simulink model "simulation_environment_thesis"  (R2024b)
settings: Solver=VariableStepAuto  StartTime=0.0  StopTime=3200  RelTol=1e-3
values from neighbouring files (symbols this model uses):
  A = [1, dt; 0, 1]    [estimate_and_control_fully_actuated_with_current.m]
  A1 = [1, 0, 0, 0, dt, 0 ... (first row only)    [estimate_and_control_fully_actuated_with_current.m]
  alpha = 10    [estimate_and_control_fully_actuated_with_current.m]
  alpha_xy_1 = 1    [estimate_and_control_fully_actuated_with_current.m]
  alpha_z_1 = 5    [estimate_and_control_fully_actuated_with_current.m]
  Az1 = [1, dt ... (first row only)    [estimate_and_control_fully_actuated_with_current.m]
  B = [dt; 0]    [estimate_and_control_fully_actuated_with_current.m]
  B1 = [dt, 0 ... (first row only)    [estimate_and_control_fully_actuated_with_current.m]
  Bz1 = [dt ... (first row only)    [estimate_and_control_fully_actuated_with_current.m]
  C1 = [1, 0, 0, 0, 0, 0 ... (first row only)    [estimate_and_control_fully_actuated_with_current.m]
  circumference_x = min_dist_from_ds*cos(theta)    [estimate_and_control_fully_actuated_with_current.m]
  circumference_y = min_dist_from_ds*sin(theta)    [estimate_and_control_fully_actuated_with_current.m]
  const_current = [-r; -s; 0; 0]    [estimate_and_control_fully_actuated_with_current.m]
  csi_psi_1 = 0.0003    [estimate_and_control_fully_actuated_with_current.m]
  csi_r_1 = 0.0003    [estimate_and_control_fully_actuated_with_current.m]
  csi_vcx_1 = 0.0003    [estimate_and_control_fully_actuated_with_current.m]
  csi_vcy_1 = 0.0003    [estimate_and_control_fully_actuated_with_current.m]
  csi_vcz_1 = 0.005    [estimate_and_control_fully_actuated_with_current.m]
  csi_x_1 = 0.003    [estimate_and_control_fully_actuated_with_current.m]
  csi_y_1 = 0.003    [estimate_and_control_fully_actuated_with_current.m]
  csi_z_1 = 0.005    [estimate_and_control_fully_actuated_with_current.m]
  Cz1 = [1, 0]    [estimate_and_control_fully_actuated_with_current.m]
  d = 1    [estimate_and_control_fully_actuated_with_current.m]
  delta_FOV = pi/7    (= 0.448798950513)    [estimate_and_control_fully_actuated_with_current.m]
  desired_speed = speed_0    [estimate_and_control_fully_actuated_with_current.m]
  dt = 1/Tsim    (= 0.005)    [estimate_and_control_fully_actuated_with_current.m]
  eta1 = deg2rad(0.5)    [estimate_and_control_fully_actuated_with_current.m]
  eta2 = deg2rad(0.5)    [estimate_and_control_fully_actuated_with_current.m]
  eta_psi_1 = 0.003    [estimate_and_control_fully_actuated_with_current.m]
  eta_x_1 = 0.03    [estimate_and_control_fully_actuated_with_current.m]
  eta_y_1 = 0.03    [estimate_and_control_fully_actuated_with_current.m]
  eta_z_1 = 0.005    [estimate_and_control_fully_actuated_with_current.m]
  G = dt*eye(2)    [estimate_and_control_fully_actuated_with_current.m]
  G1 = dt*eye(6)    [estimate_and_control_fully_actuated_with_current.m]
  Gz1 = dt*eye(2)    [estimate_and_control_fully_actuated_with_current.m]
  left_reference = L_x*[cos(NED_psi_ds); sin(NED_psi_ds); 0] + L_y_l*[-sin(NED_psi_ds); cos(NED_psi_ds); 0]    [estimate_and_control_fully_actuated_with_current.m]
  max_dist_from_ship = 60    [estimate_and_control_fully_actuated_with_current.m]
  min_dist_from_ds = 45    [estimate_and_control_fully_actuated_with_current.m]
  mu = -5    [estimate_and_control_fully_actuated_with_current.m]
  NED_psi_ds = -pi/2    (= -1.57079632679)    [estimate_and_control_fully_actuated_with_current.m]
  p_b1 = [-90; 90; 5]    [estimate_and_control_fully_actuated_with_current.m]
  position_t_0 = [0; 0; 0; 0]    [estimate_and_control_fully_actuated_with_current.m]
  psi_P = linspace(0,2*pi,360)'    [estimate_and_control_fully_actuated_with_current.m]
  Q = [csi1_kf^2, 0 ... (first row only)    [estimate_and_control_fully_actuated_with_current.m]
  Q1 = [csi_x_1_kf^2, 0, 0, 0, 0, 0 ... (first row only)    [estimate_and_control_fully_actuated_with_current.m]
  Qz1 = [csi_z_1_kf^2, 0 ... (first row only)    [estimate_and_control_fully_actuated_with_current.m]
  R = [eta1_kf^2, 0 ... (first row only)    [estimate_and_control_fully_actuated_with_current.m]
  R1 = [eta_x_1_kf^2, 0, 0 ... (first row only)    [estimate_and_control_fully_actuated_with_current.m]
  right_reference = L_x*[cos(NED_psi_ds); sin(NED_psi_ds); 0] + L_y_r*[sin(NED_psi_ds); -cos(NED_psi_ds); 0]    [estimate_and_control_fully_actuated_with_current.m]
  Rz1 = eta_z_1_kf^2    (= 1e-06)    [estimate_and_control_fully_actuated_with_current.m]
  speed_0 = [0.1, 0.1, 0, 0]'    [estimate_and_control_fully_actuated_with_current.m]
  tau_heave_sat = 30    [estimate_and_control_fully_actuated_with_current.m]
  tau_surge_sat = 10    [estimate_and_control_fully_actuated_with_current.m]
  tau_sway_sat = 30    [estimate_and_control_fully_actuated_with_current.m]
  tau_yaw_sat = 0.1    [estimate_and_control_fully_actuated_with_current.m]
  var_vm_x = 0.001    [estimate_and_control_fully_actuated_with_current.m]
  var_vm_y = 0.001    [estimate_and_control_fully_actuated_with_current.m]
  var_vm_z = 0.001    [estimate_and_control_fully_actuated_with_current.m]
undefined symbols (not defined in the file or in its folder): A2, alpha2, B2, csi1_2, csi2_2, csi3_2, csi4_2, csi5_2, csi6_2, csi7_2, csi8_2, eta1_2, eta2_2, eta3_2, eta4_2, eta5_2, eta6_2, G2, Q2, R2
libraries: simulink x60, simulink_extras x5
(part 2 of 16)

== system simulation_environment_thesis/PHASE 2.22 ==
  note: i know the beacon is my new
      | reference frame, so (0,0,0)
  note: i know the beacon is my new
      | reference frame, so (0,0,0)
  note: true position to plot the data in matlab
  note: physical data to be sent to
      | the range-measurement simulator
  note: ship_psi_auv
  note: ok, trasformazione coerente
  note: ok cominciano esattamente dalla flag
  ship_psi_auv : Inport
  ship_p_auv : Inport  Port=2
  ship_p_ds : Inport  Port=3
  Enable : EnablePort
  CONTROLLER4 : Subsystem
  Constant : Constant  Value=0
  Constant1 : Constant  Value=min_dist_from_ds
  Constant9 : Constant  Value=desired_speed
  Data Store Read : DataStoreRead  DataStoreName=x_hat_12_xy_3  IndexOptions=Index vector (dialog)  Indices=1  OutputSizes=1
  Data Store Read1 : DataStoreRead  DataStoreName=x_hat_12_z_3  IndexOptions=Index vector (dialog)  Indices=1  OutputSizes=1
  Data Store Read2 : DataStoreRead  DataStoreName=P0_12_xy_3  IndexOptions=Index vector (dialog)  Indices=1  OutputSizes=1
  Data Store Read3 : DataStoreRead  DataStoreName=P0_12_z_3  IndexOptions=Index vector (dialog)  Indices=1  OutputSizes=1
  Data Store Write : DataStoreWrite  DataStoreName=x_hat_23_3  IndexOptions=Index vector (dialog)  Indices=1  OutputSizes=1
  Data Store Write1 : DataStoreWrite  DataStoreName=P0_23_3  IndexOptions=Index vector (dialog)  Indices=1  OutputSizes=1
  MATLAB Function : MATLAB Function  TreatAsAtomicUnit=on
  MATLAB Function1 : MATLAB Function  TreatAsAtomicUnit=on
  MATLAB Function2 : MATLAB Function  TreatAsAtomicUnit=on
  MATLAB Function7 : MATLAB Function  TreatAsAtomicUnit=on
  Memory2 : Memory
  Scope{,1..3} (4 alike) : Scope
  Selector1 : Selector  InputPortWidth=4  IndexOptions=Index vector (dialog)  Indices=[1 2 3]  OutputSizes=1
  Selector2 : Selector  InputPortWidth=3  IndexOptions=Index vector (dialog)  Indices=[3]  OutputSizes=1
  Selector3 : Selector  InputPortWidth=3  IndexOptions=Index vector (dialog)  Indices=[1 2]  OutputSizes=1
  Selector4 : Selector  InputPortWidth=8  IndexOptions=Index vector (dialog)  Indices=[1 2 7 8 4 5]  OutputSizes=1
  Selector5 : Selector  InputPortWidth=8  IndexOptions=Index vector (dialog)  Indices=[3 6]  OutputSizes=1
  Sum : Sum  Inputs=|+-
  Sum1 : Sum  Inputs=|+-
  TAU : Scope
  To Workspace : ToWorkspace  VariableName=switchposition12  SampleTime=-1
  dynamic + kinematic models1 : Subsystem
  new origin : Constant  Value=[0; 0]  VectorParams1D=off
  "p, v_c, psi, r (3D) estimate, from RANGE, v_c, PSI measurement" : Subsystem
  v error (robot frame)5 : Scope
  yaw_err3 : Scope
  z_err : Scope
  s_p_auv : Outport
  s_psi_auv : Outport  Port=2
  stop_flag_2 : Outport  Port=3
  ds_p_auv : Outport  Port=4
  body speed : Outport  Port=5
  state_est_plane : Outport  Port=6
  state_est_z : Outport  Port=7
  angle : Outport  Port=8
  error speed : Outport  Port=9
  z_ err : Outport  Port=10
  wires:
  "p, v_c, psi, r (3D) estimate, from RANGE, v_c, PSI measurement"/2(psi_est) -> dynamic + kinematic models1/4(yaw_est), Sum1/2
  Constant9/1 -> dynamic + kinematic models1/2(ref speed), MATLAB Function7/5(speed), CONTROLLER4/3(speed_0)
  CONTROLLER4/1(tau) -> dynamic + kinematic models1/1(tau), TAU/1
  dynamic + kinematic models1/1(error speed) -> CONTROLLER4/1(v_err), error speed/1, v error (robot frame)5/1
  ship_psi_auv/1 -> dynamic + kinematic models1/3(last_yaw__from_ship)
  ship_p_ds/1 -> MATLAB Function1/2(ship_p_ds), MATLAB Function/2(ship_p_ds)
  dynamic + kinematic models1/3(p) -> Selector1/1
  MATLAB Function1/1(ship_p_auv) -> s_p_auv/1
  dynamic + kinematic models1/4(yaw) -> s_psi_auv/1, "p, v_c, psi, r (3D) estimate, from RANGE, v_c, PSI measurement"/3(psi)
  ship_p_auv/1 -> To Workspace/1, MATLAB Function/1(ship_p_auv)
  Sum1/1 -> MATLAB Function2/1("angle ")
  dynamic + kinematic models1/2(v_tot ds) -> "p, v_c, psi, r (3D) estimate, from RANGE, v_c, PSI measurement"/2(inert_vel)
  Sum/1 -> CONTROLLER4/4(z_err), z_ err/1, z_err/1
  Constant/1 -> Sum/1
  new origin/1 -> MATLAB Function7/4(beacon)
  Constant1/1 -> MATLAB Function7/6(threshold)
  "p, v_c, psi, r (3D) estimate, from RANGE, v_c, PSI measurement"/3(v_c_xyz) -> MATLAB Function7/2(v_c)
  MATLAB Function7/1(yaw_desired) -> Scope3/1, Sum1/1
  MATLAB Function7/2(stop_flag) -> Memory2/1, Scope/1, stop_flag_2/1
  MATLAB Function7/3(ds_dist) -> Scope2/1
  MATLAB Function2/1(angle) -> angle/1, CONTROLLER4/2(psi_err), yaw_err3/1
  Memory2/1 -> MATLAB Function7/1("stop_flag ")
  "p, v_c, psi, r (3D) estimate, from RANGE, v_c, PSI measurement"/1(xyz_est) -> Selector2/1, Selector3/1
  Selector2/1 -> Sum/2
  Selector3/1 -> MATLAB Function7/3(p)
  dynamic + kinematic models1/5(body speed) -> body speed/1
  MATLAB Function/1(ds_p_auv) -> dynamic + kinematic models1/5(ds_p_auv), Scope1/1
  Selector1/1 -> ds_p_auv/1, MATLAB Function1/1(ds_p_auv), "p, v_c, psi, r (3D) estimate, from RANGE, v_c, PSI measurement"/1(p)
  Data Store Read/1 -> "p, v_c, psi, r (3D) estimate, from RANGE, v_c, PSI measurement"/4(x_0_hat_xy)
  Data Store Read1/1 -> "p, v_c, psi, r (3D) estimate, from RANGE, v_c, PSI measurement"/5(x_0_hat_z)
  Data Store Read2/1 -> "p, v_c, psi, r (3D) estimate, from RANGE, v_c, PSI measurement"/6(P0_xy)
  Data Store Read3/1 -> "p, v_c, psi, r (3D) estimate, from RANGE, v_c, PSI measurement"/7(P0_z)
  "p, v_c, psi, r (3D) estimate, from RANGE, v_c, PSI measurement"/5(P023) -> Data Store Write1/1
  Selector5/1 -> state_est_z/1
  Selector4/1 -> state_est_plane/1
  "p, v_c, psi, r (3D) estimate, from RANGE, v_c, PSI measurement"/4(state_est) -> Selector5/1, Selector4/1, Data Store Write/1

== system simulation_environment_thesis/PHASE 2.24 ==
  note: ds_psi_auv
  note: ok, trasformazione coerente
  note: ok cominciano esattamente dalla flag
  ship_psi_auv : Inport
  ship_p_auv : Inport  Port=2
  ship_p_ds : Inport  Port=3
  Enable : EnablePort
  CONTROLLER4 : Subsystem
  Constant : Constant  Value=0
  Constant1 : Constant  Value=min_dist_from_ds
  Constant9 : Constant  Value=desired_speed
  Data Store Read : DataStoreRead  DataStoreName=x_hat_12_xy_1  IndexOptions=Index vector (dialog)  Indices=1  OutputSizes=1
  Data Store Read1 : DataStoreRead  DataStoreName=P0_12_xy_1  IndexOptions=Index vector (dialog)  Indices=1  OutputSizes=1
  Data Store Read2 : DataStoreRead  DataStoreName=x_hat_12_z_1  IndexOptions=Index vector (dialog)  Indices=1  OutputSizes=1
  Data Store Read3 : DataStoreRead  DataStoreName=P0_12_z_1  IndexOptions=Index vector (dialog)  Indices=1  OutputSizes=1
  Data Store Write : DataStoreWrite  DataStoreName=x_hat_23_xy_1  IndexOptions=Index vector (dialog)  Indices=1  OutputSizes=1
  Data Store Write1 : DataStoreWrite  DataStoreName=P0_23_xy_1  IndexOptions=Index vector (dialog)  Indices=1  OutputSizes=1
  Data Store Write2 : DataStoreWrite  DataStoreName=x_hat_23_z_1  IndexOptions=Index vector (dialog)  Indices=1  OutputSizes=1
  Data Store Write3 : DataStoreWrite  DataStoreName=P0_23_z_1  IndexOptions=Index vector (dialog)  Indices=1  OutputSizes=1
  MATLAB Function : MATLAB Function  TreatAsAtomicUnit=on
  MATLAB Function1 : MATLAB Function  TreatAsAtomicUnit=on
  MATLAB Function2 : MATLAB Function  TreatAsAtomicUnit=on
  MATLAB Function7 : MATLAB Function  TreatAsAtomicUnit=on
  Memory2 : Memory
  Scope1 : Scope
  Scope2 : Scope
  Scope3 : Scope
  Selector1 : Selector  InputPortWidth=4  IndexOptions=Index vector (dialog)  Indices=[1 2 3]  OutputSizes=1
  Sum : Sum  Inputs=|+-
  Sum1 : Sum  Inputs=|+-
  TAU : Scope
  To Workspace : ToWorkspace  VariableName=switchposition12  SampleTime=-1
  dynamic + kinematic models1 : Subsystem
  new origin : Constant  Value=[0; 0]  VectorParams1D=off
  "positon and current estimate, XY PLANE from X, Y, psi measurement" : Subsystem
  v error (robot frame)5 : Scope
  yaw_err3 : Scope
  "z, v_c_z estimate from DEPTH measurement" : Subsystem
  z_err : Scope
  speed body : Outport
  s_p_auv : Outport  Port=2
  s_psi_auv : Outport  Port=3
  stop_flag_2 : Outport  Port=4
  ds_p_auv : Outport  Port=5
  state_est_plane : Outport  Port=6
  state_est_z : Outport  Port=7
  error speed : Outport  Port=8
  angle : Outport  Port=9
  "z_err " : Outport  Port=10
  wires:
  "positon and current estimate, XY PLANE from X, Y, psi measurement"/2(psi_est) -> dynamic + kinematic models1/4(yaw_est), Sum1/2
  Constant9/1 -> dynamic + kinematic models1/2(ref speed), CONTROLLER4/3(speed_0), MATLAB Function7/5(speed)
  CONTROLLER4/1(tau) -> dynamic + kinematic models1/1(tau), TAU/1
  dynamic + kinematic models1/1(error speed) -> error speed/1, CONTROLLER4/1(v_err), v error (robot frame)5/1
  MATLAB Function/1(ds_p_auv) -> dynamic + kinematic models1/5(ds_p_auv), Scope1/1
  ship_psi_auv/1 -> dynamic + kinematic models1/3(last_yaw__from_ship)
  ship_p_ds/1 -> MATLAB Function1/2(ship_p_ds), MATLAB Function/2(ship_p_ds)
  dynamic + kinematic models1/4(p) -> Selector1/1, "positon and current estimate, XY PLANE from X, Y, psi measurement"/1(p)
  MATLAB Function1/1(ship_p_auv) -> s_p_auv/1
  dynamic + kinematic models1/5(yaw) -> s_psi_auv/1, "positon and current estimate, XY PLANE from X, Y, psi measurement"/3(psi)
  ship_p_auv/1 -> To Workspace/1, MATLAB Function/1(ship_p_auv)
  Sum1/1 -> MATLAB Function2/1("angle ")
  dynamic + kinematic models1/2(v_tot ds) -> "positon and current estimate, XY PLANE from X, Y, psi measurement"/2(inert_vel)
  dynamic + kinematic models1/3(body speed) -> speed body/1
  "z, v_c_z estimate from DEPTH measurement"/1(z_est) -> Sum/2
  Sum/1 -> z_err/1, "z_err "/1, CONTROLLER4/4(z_err)
  Constant/1 -> Sum/1
  new origin/1 -> MATLAB Function7/4(beacon)
  Constant1/1 -> MATLAB Function7/6(threshold)
  "positon and current estimate, XY PLANE from X, Y, psi measurement"/3(v_c_xy) -> MATLAB Function7/2(v_c)
  MATLAB Function7/1(yaw_desired) -> Scope3/1, Sum1/1
  MATLAB Function7/2(stop_flag) -> Memory2/1, stop_flag_2/1
  MATLAB Function7/3(ds_dist) -> Scope2/1
  MATLAB Function2/1(angle) -> angle/1, CONTROLLER4/2(psi_err), yaw_err3/1
  Memory2/1 -> MATLAB Function7/1("stop_flag ")
  "positon and current estimate, XY PLANE from X, Y, psi measurement"/1(xy_est) -> MATLAB Function7/3(p)
  Data Store Read/1 -> "positon and current estimate, XY PLANE from X, Y, psi measurement"/4(x_0_hat_xy)
  Data Store Read1/1 -> "positon and current estimate, XY PLANE from X, Y, psi measurement"/5(P0_xy)
  "positon and current estimate, XY PLANE from X, Y, psi measurement"/4(state_est) -> state_est_plane/1, Data Store Write/1
  "positon and current estimate, XY PLANE from X, Y, psi measurement"/5(P0) -> Data Store Write1/1
  Selector1/1 -> ds_p_auv/1, "z, v_c_z estimate from DEPTH measurement"/1(p), MATLAB Function1/1(ds_p_auv)
  "z, v_c_z estimate from DEPTH measurement"/2(state_est) -> state_est_z/1, Data Store Write2/1
  "z, v_c_z estimate from DEPTH measurement"/3(P0) -> Data Store Write3/1
  Data Store Read2/1 -> "z, v_c_z estimate from DEPTH measurement"/2(x_0_hat_z)
  Data Store Read3/1 -> "z, v_c_z estimate from DEPTH measurement"/3(P0_z)

== system simulation_environment_thesis/PHASE 3&4   ==
  note: from phase 2 to either
      | phase 3 or directly phase 4
  note: FASE 4
  ship_p_ds : Inport
  ds_p_auv : Inport  Port=2
  stop_flag : Inport  Port=3
  ship_psi_auv : Inport  Port=4
  ship_p_auv : Inport  Port=5
  Enable : EnablePort
  AND1 : Logic  IconShape=distinctive  AllPortsSameDT=off  OutDataTypeStr=boolean
  AND2 : Logic  IconShape=distinctive  AllPortsSameDT=off  OutDataTypeStr=boolean
  NOT3 : Logic  Operator=NOT  IconShape=distinctive  AllPortsSameDT=off  OutDataTypeStr=boolean
  NOT5 : Logic  Operator=NOT  IconShape=distinctive  AllPortsSameDT=off  OutDataTypeStr=boolean
  NOT6 : Logic  Operator=NOT  IconShape=distinctive  AllPortsSameDT=off  OutDataTypeStr=boolean
  OR : Logic  Operator=OR  IconShape=distinctive  AllPortsSameDT=off  OutDataTypeStr=boolean
  PHASE  3 : Subsystem
  PHASE  4 : Subsystem
  Scope{3..4,10..12} (5 alike) : Scope
  Subsystem3 : Subsystem
  Switch : Switch  Criteria=u2 > Threshold  SaturateOnIntegerOverflow=off  InputSameDT=off
  Switch1 : Switch  Criteria=u2 > Threshold  SaturateOnIntegerOverflow=off  InputSameDT=off
  To Workspace : ToWorkspace  VariableName=pass_by_3  SampleTime=-1
  Unit Delay4 : UnitDelay  InitialCondition=1  SampleTime=-1  HasFrameUpgradeWarning=on
  Unit Delay5 : UnitDelay  InitialCondition=1  SampleTime=-1  HasFrameUpgradeWarning=on
  end phase 3 : Subsystem
  "logic to recognize where i am, based on the # of pings" : Subsystem
  simulator of pings from sensor : Subsystem
  speed body : Outport
  ship_psi_auv1 : Outport  Port=2
  ship_p_auv1 : Outport  Port=3
  speed body1 : Outport  Port=4
  ship_psi_auv2 : Outport  Port=5
  ship_p_auv2 : Outport  Port=6
  out : Outport  Port=7
  out1 : Outport  Port=8
  docked : Outport  Port=9
  ds_p_auv_3 : Outport  Port=10
  state_est_plane4 : Outport  Port=11
  state_est_z3 : Outport  Port=12
  state_est_plane3 : Outport  Port=13
  state_est_z4 : Outport  Port=14
  ds_p_auv4 : Outport  Port=15
  z_ err : Outport  Port=16
  yaw_error : Outport  Port=17
  error speed : Outport  Port=18
  z_ err 4 : Outport  Port=19
  angle 4 : Outport  Port=20
  error speed 4 : Outport  Port=21
  wires:
  Unit Delay5/1 -> AND2/2
  PHASE  3/4(ds_p_auv) -> ds_p_auv_3/1, end phase 3/1(ds_p_auv)
  Subsystem3/1(out) -> out1/1, Scope12/1, OR/1, NOT5/1
  PHASE  4/2(s_p_auv) -> ship_p_auv2/1
  ship_p_ds/1 -> PHASE  4/3(ship_p_ds), PHASE  3/3(ship_p_ds)
  "logic to recognize where i am, based on the # of pings"/1(out) -> PHASE  4/4(direct_to_4), Switch1/2, Switch/2, out/1, NOT3/1, To Workspace/1, Scope4/1, OR/2
  ship_psi_auv/1 -> Switch/1, PHASE  3/1(ship_psi_auv)
  Unit Delay4/1 -> AND1/2
  PHASE  4/4(docked) -> docked/1, NOT6/1, Scope3/1
  simulator of pings from sensor/1(# OF PINGS) -> "logic to recognize where i am, based on the # of pings"/1(num)
  NOT3/1 -> Subsystem3/Enable, end phase 3/Enable, AND1/1
  AND1/1 -> PHASE  3/Enable
  OR/1 -> Scope11/1, AND2/1
  PHASE  3/2(s_p_auv) -> Switch1/3, ship_p_auv1/1
  PHASE  4/3(s_psi_auv) -> ship_psi_auv2/1
  NOT5/1 -> Unit Delay4/1
  PHASE  3/3(s_psi_auv) -> Switch/3, ship_psi_auv1/1
  end phase 3/1(num_of_pings) -> Subsystem3/1(num)
  AND2/1 -> Scope10/1, PHASE  4/Enable
  stop_flag/1 -> simulator of pings from sensor/2(stop_flag)
  ds_p_auv/1 -> simulator of pings from sensor/1(ds_p_auv)
  ship_p_auv/1 -> Switch1/1, PHASE  3/2(ship_p_auv)
  PHASE  4/1(speed body) -> speed body1/1
  PHASE  3/1(speed body) -> speed body/1
  NOT6/1 -> Unit Delay5/1
  Switch/1 -> PHASE  4/1(ship_psi_auv)
  Switch1/1 -> PHASE  4/2(ship_p_auv)
  PHASE  3/5(state_est_plane) -> state_est_plane3/1
  PHASE  3/6(state_est_z) -> state_est_z3/1
  PHASE  4/7(ds_p_auv) -> ds_p_auv4/1
  PHASE  4/6(state_est_z) -> state_est_z4/1
  PHASE  4/5(state_est_plane) -> state_est_plane4/1
  PHASE  3/7(z_ err) -> z_ err/1
  PHASE  3/8(yaw_error) -> yaw_error/1
  PHASE  3/9(error speed) -> error speed/1
  PHASE  4/8(z_ err) -> z_ err 4/1
  PHASE  4/9(angle) -> angle 4/1
  PHASE  4/10(error speed) -> error speed 4/1

== system simulation_environment_thesis/PHASE 3&4.21 ==
  note: from phase 2 to either phase 3 or directly phase 4
  note: FASE 4
  ship_p_ds : Inport
  ds_p_auv : Inport  Port=2
  stop_flag : Inport  Port=3
  ship_psi_auv : Inport  Port=4
  ship_p_auv : Inport  Port=5
  Enable : EnablePort
  AND1 : Logic  IconShape=distinctive  AllPortsSameDT=off  OutDataTypeStr=boolean
  AND2 : Logic  IconShape=distinctive  AllPortsSameDT=off  OutDataTypeStr=boolean
  NOT3 : Logic  Operator=NOT  IconShape=distinctive  AllPortsSameDT=off  OutDataTypeStr=boolean
  NOT5 : Logic  Operator=NOT  IconShape=distinctive  AllPortsSameDT=off  OutDataTypeStr=boolean
  NOT6 : Logic  Operator=NOT  IconShape=distinctive  AllPortsSameDT=off  OutDataTypeStr=boolean
  OR : Logic  Operator=OR  IconShape=distinctive  AllPortsSameDT=off  OutDataTypeStr=boolean
  PHASE  3 : Subsystem
  PHASE  4 : Subsystem
  Scope{3..4,8,10..12} (6 alike) : Scope
  Subsystem3 : Subsystem
  Switch : Switch  Criteria=u2 > Threshold  SaturateOnIntegerOverflow=off  InputSameDT=off
  Switch1 : Switch  Criteria=u2 > Threshold  SaturateOnIntegerOverflow=off  InputSameDT=off
  To Workspace : ToWorkspace  VariableName=pass_by_3  SampleTime=-1
  Unit Delay4 : UnitDelay  InitialCondition=1  SampleTime=-1  HasFrameUpgradeWarning=on
  Unit Delay5 : UnitDelay  InitialCondition=1  SampleTime=-1  HasFrameUpgradeWarning=on
  end phase 3 : Subsystem
  "logic to recognize where i am, based on the # of pings" : Subsystem
  simulator of pings from sensor : Subsystem
  speed body : Outport
  ship_psi_auv1 : Outport  Port=2
  ship_p_auv1 : Outport  Port=3
  speed body1 : Outport  Port=4
  ship_psi_auv2 : Outport  Port=5
  ship_p_auv2 : Outport  Port=6
  out : Outport  Port=7
  out1 : Outport  Port=8
  docked : Outport  Port=9
  state_est_plane_3 : Outport  Port=10
  state_est_z_3 : Outport  Port=11
  state_est_plane_4 : Outport  Port=12
  state_est_z_4 : Outport  Port=13
  ds_p_auv_3 : Outport  Port=14
  ds_p_auv_4 : Outport  Port=15
  "z_err " : Outport  Port=16
  error speed : Outport  Port=17
  yaw_error : Outport  Port=18
  z_err 4 : Outport  Port=19
  angle 4 : Outport  Port=20
  error speed 4 : Outport  Port=21
  wires:
  Unit Delay5/1 -> AND2/2
  PHASE  3/4(ds_p_auv) -> end phase 3/1(ds_p_auv), ds_p_auv_3/1
  Subsystem3/1(out) -> out1/1, Scope12/1, OR/1, NOT5/1
  PHASE  4/2(s_p_auv) -> ship_p_auv2/1
  ship_p_ds/1 -> PHASE  4/3(ship_p_ds), PHASE  3/3(ship_p_ds)
  "logic to recognize where i am, based on the # of pings"/1(out) -> PHASE  4/4(direct_to_4), Switch1/2, Switch/2, out/1, NOT3/1, To Workspace/1, Scope4/1, OR/2
  ship_psi_auv/1 -> Switch/1, PHASE  3/1(ship_psi_auv)
  PHASE  4/4(new_speed_desired) -> Scope8/1
  Unit Delay4/1 -> AND1/2
  PHASE  4/5(docked) -> docked/1, NOT6/1, Scope3/1
  simulator of pings from sensor/1(# OF PINGS) -> "logic to recognize where i am, based on the # of pings"/1(num)
  NOT3/1 -> Subsystem3/Enable, end phase 3/Enable, AND1/1
  AND1/1 -> PHASE  3/Enable
  OR/1 -> Scope11/1, AND2/1
  PHASE  3/2(s_p_auv) -> Switch1/3, ship_p_auv1/1
  PHASE  4/3(s_psi_auv) -> ship_psi_auv2/1
  NOT5/1 -> Unit Delay4/1
  PHASE  3/3(s_psi_auv) -> Switch/3, ship_psi_auv1/1
  end phase 3/1(num_of_pings) -> Subsystem3/1(num)
  AND2/1 -> Scope10/1, PHASE  4/Enable
  stop_flag/1 -> simulator of pings from sensor/2(stop_flag)
  ds_p_auv/1 -> simulator of pings from sensor/1(ds_p_auv)
  ship_p_auv/1 -> Switch1/1, PHASE  3/2(ship_p_auv)
  PHASE  4/1(speed body) -> speed body1/1
  PHASE  3/1(speed body) -> speed body/1
  NOT6/1 -> Unit Delay5/1
  Switch/1 -> PHASE  4/1(ship_psi_auv)
  Switch1/1 -> PHASE  4/2(ship_p_auv)
  PHASE  3/5(state_est_plane) -> state_est_plane_3/1
  PHASE  3/6(state_est_z) -> state_est_z_3/1
  PHASE  4/6(state_est_plane) -> state_est_plane_4/1
  PHASE  4/7(state_est_z) -> state_est_z_4/1
  PHASE  4/8(ds_p_auv_4) -> ds_p_auv_4/1
  PHASE  3/7("z_err ") -> "z_err "/1
  PHASE  3/8(error speed) -> error speed/1
  PHASE  3/9(yaw_error) -> yaw_error/1
  PHASE  4/9(z_ err) -> z_err 4/1
  PHASE  4/10(angle) -> angle 4/1
  PHASE  4/11(error speed) -> error speed 4/1

== system simulation_environment_thesis/PHASE 3&4.22 ==
  note: from phase 2 to either phase 3 or directly phase 4
  note: FASE 4
  ship_p_ds : Inport
  ds_p_auv : Inport  Port=2
  stop_flag : Inport  Port=3
  ship_psi_auv : Inport  Port=4
  ship_p_auv : Inport  Port=5
  Enable : EnablePort
  AND1 : Logic  IconShape=distinctive  AllPortsSameDT=off  OutDataTypeStr=boolean
  AND2 : Logic  IconShape=distinctive  AllPortsSameDT=off  OutDataTypeStr=boolean
  NOT3 : Logic  Operator=NOT  IconShape=distinctive  AllPortsSameDT=off  OutDataTypeStr=boolean
  NOT5 : Logic  Operator=NOT  IconShape=distinctive  AllPortsSameDT=off  OutDataTypeStr=boolean
  NOT6 : Logic  Operator=NOT  IconShape=distinctive  AllPortsSameDT=off  OutDataTypeStr=boolean
  OR : Logic  Operator=OR  IconShape=distinctive  AllPortsSameDT=off  OutDataTypeStr=boolean
  PHASE  3 : Subsystem
  PHASE  4 : Subsystem
  Scope{3..4,8,10..12} (6 alike) : Scope
  Subsystem3 : Subsystem
  Switch : Switch  Criteria=u2 > Threshold  SaturateOnIntegerOverflow=off  InputSameDT=off
  Switch1 : Switch  Criteria=u2 > Threshold  SaturateOnIntegerOverflow=off  InputSameDT=off
  To Workspace : ToWorkspace  VariableName=pass_by_3  SampleTime=-1
  Unit Delay4 : UnitDelay  InitialCondition=1  SampleTime=-1  HasFrameUpgradeWarning=on
  Unit Delay5 : UnitDelay  InitialCondition=1  SampleTime=-1  HasFrameUpgradeWarning=on
  end phase 3 : Subsystem
  "logic to recognize where i am, based on the # of pings" : Subsystem
  simulator of pings from sensor : Subsystem
  speed body : Outport
  ship_psi_auv1 : Outport  Port=2
  ship_p_auv1 : Outport  Port=3
  speed body1 : Outport  Port=4
  ship_psi_auv2 : Outport  Port=5
  ship_p_auv2 : Outport  Port=6
  out : Outport  Port=7
  out1 : Outport  Port=8
  docked : Outport  Port=9
  ds_p_auv_3 : Outport  Port=10
  ds_p_auv4 : Outport  Port=11
  state_est_plane4 : Outport  Port=12
  state_est_z4 : Outport  Port=13
  state_est_plane3 : Outport  Port=14
  state_est_z3 : Outport  Port=15
  yaw_rel_err : Outport  Port=16
  error speed : Outport  Port=17
  yaw_error : Outport  Port=18
  z_ err : Outport  Port=19
  error speed 4 : Outport  Port=20
  angle 4 : Outport  Port=21
  "z_ err 4 " : Outport  Port=22
  wires:
  Unit Delay5/1 -> AND2/2
  PHASE  3/4(ds_p_auv) -> end phase 3/1(ds_p_auv), ds_p_auv_3/1
  Subsystem3/1(out) -> out1/1, Scope12/1, OR/1, NOT5/1
  PHASE  4/2(s_p_auv) -> ship_p_auv2/1
  ship_p_ds/1 -> PHASE  4/3(ship_p_ds), PHASE  3/3(ship_p_ds)
  "logic to recognize where i am, based on the # of pings"/1(out) -> PHASE  4/4(direct_to_4), Switch1/2, Switch/2, out/1, NOT3/1, To Workspace/1, Scope4/1, OR/2
  ship_psi_auv/1 -> Switch/1, PHASE  3/1(ship_psi_auv)
  PHASE  4/4(new_speed_desired) -> Scope8/1
  Unit Delay4/1 -> AND1/2
  PHASE  4/5(docked) -> NOT6/1, Scope3/1, docked/1
  simulator of pings from sensor/1(# OF PINGS) -> "logic to recognize where i am, based on the # of pings"/1(num)
  NOT3/1 -> Subsystem3/Enable, end phase 3/Enable, AND1/1
  AND1/1 -> PHASE  3/Enable
  OR/1 -> Scope11/1, AND2/1
  PHASE  3/2(s_p_auv) -> Switch1/3, ship_p_auv1/1
  PHASE  4/3(s_psi_auv) -> ship_psi_auv2/1
  NOT5/1 -> Unit Delay4/1
  PHASE  3/3(s_psi_auv) -> Switch/3, ship_psi_auv1/1
  end phase 3/1(num_of_pings) -> Subsystem3/1(num)
  AND2/1 -> Scope10/1, PHASE  4/Enable
  stop_flag/1 -> simulator of pings from sensor/2(stop_flag)
  ds_p_auv/1 -> simulator of pings from sensor/1(ds_p_auv)
  ship_p_auv/1 -> Switch1/1, PHASE  3/2(ship_p_auv)
  PHASE  4/1(speed body) -> speed body1/1
  PHASE  3/1(speed body) -> speed body/1
  NOT6/1 -> Unit Delay5/1
  Switch/1 -> PHASE  4/1(ship_psi_auv)
  Switch1/1 -> PHASE  4/2(ship_p_auv)
  PHASE  3/6(state_est_z) -> state_est_z3/1
  PHASE  3/5(state_est_plane) -> state_est_plane3/1
  PHASE  4/6(ds_p_auv4) -> ds_p_auv4/1
  PHASE  4/7(state_est_plane4) -> state_est_plane4/1
  PHASE  4/8(state_est_z) -> state_est_z4/1
  PHASE  4/9(yaw_rel_err) -> yaw_rel_err/1
  PHASE  3/7(error speed) -> error speed/1
  PHASE  3/8(yaw_error) -> yaw_error/1
  PHASE  3/9(z_ err) -> z_ err/1
  PHASE  4/10(error speed) -> error speed 4/1
  PHASE  4/11(angle) -> angle 4/1
  PHASE  4/12("z_ err ") -> "z_ err 4 "/1

== system simulation_environment_thesis/PHASE 3&4.24 ==
  note: from phase 2 to either phase 3 or directly phase 4
  note: FASE 4
  ship_p_ds : Inport
  ds_p_auv : Inport  Port=2
  stop_flag : Inport  Port=3
  ship_psi_auv : Inport  Port=4
  ship_p_auv : Inport  Port=5
  Enable : EnablePort
  AND1 : Logic  IconShape=distinctive  AllPortsSameDT=off  OutDataTypeStr=boolean
  AND2 : Logic  IconShape=distinctive  AllPortsSameDT=off  OutDataTypeStr=boolean
  NOT3 : Logic  Operator=NOT  IconShape=distinctive  AllPortsSameDT=off  OutDataTypeStr=boolean
  NOT5 : Logic  Operator=NOT  IconShape=distinctive  AllPortsSameDT=off  OutDataTypeStr=boolean
  NOT6 : Logic  Operator=NOT  IconShape=distinctive  AllPortsSameDT=off  OutDataTypeStr=boolean
  OR : Logic  Operator=OR  IconShape=distinctive  AllPortsSameDT=off  OutDataTypeStr=boolean
  PHASE  3 : Subsystem
  PHASE  4 : Subsystem
  Scope{3..4,8,10..12} (6 alike) : Scope
  Subsystem3 : Subsystem
  Switch : Switch  Criteria=u2 > Threshold  SaturateOnIntegerOverflow=off  InputSameDT=off
  Switch1 : Switch  Criteria=u2 > Threshold  SaturateOnIntegerOverflow=off  InputSameDT=off
  To Workspace : ToWorkspace  VariableName=pass_by_3  SampleTime=-1
  Unit Delay4 : UnitDelay  InitialCondition=1  SampleTime=-1  HasFrameUpgradeWarning=on
  Unit Delay5 : UnitDelay  InitialCondition=1  SampleTime=-1  HasFrameUpgradeWarning=on
  end phase 3 : Subsystem
  "logic to recognize where i am, based on the # of pings" : Subsystem
  simulator of pings from sensor : Subsystem
  speed body : Outport
  ship_psi_auv1 : Outport  Port=2
  ship_p_auv1 : Outport  Port=3
  speed body1 : Outport  Port=4
  ship_psi_auv2 : Outport  Port=5
  ship_p_auv2 : Outport  Port=6
  out : Outport  Port=7
  out1 : Outport  Port=8
  docked : Outport  Port=9
  state_est_plane_3 : Outport  Port=10
  state_est_z_3 : Outport  Port=11
  state_est_plane_4 : Outport  Port=12
  state_est_z_4 : Outport  Port=13
  state_est_yaw_rel_4 : Outport  Port=14
  ds_p_auv_phase3 : Outport  Port=15
  ds_p_auv_phase4 : Outport  Port=16
  yaw_error : Outport  Port=17
  "z_err " : Outport  Port=18
  error speed : Outport  Port=19
  error speed 4 : Outport  Port=20
  angle 4 : Outport  Port=21
  z err 4 : Outport  Port=22
  wires:
  Unit Delay5/1 -> AND2/2
  PHASE  3/4(ds_p_auv) -> end phase 3/1(ds_p_auv), ds_p_auv_phase3/1
  Subsystem3/1(out) -> out1/1, Scope12/1, OR/1, NOT5/1
  PHASE  4/2(s_p_auv) -> ship_p_auv2/1
  ship_p_ds/1 -> PHASE  4/3(ship_p_ds), PHASE  3/3(ship_p_ds)
  "logic to recognize where i am, based on the # of pings"/1(out) -> PHASE  4/4(direct_to_4), Switch1/2, Switch/2, out/1, NOT3/1, To Workspace/1, Scope4/1, OR/2
  ship_psi_auv/1 -> Switch/1, PHASE  3/1(ship_psi_auv)
  PHASE  4/4(new_speed_desired) -> Scope8/1
  Unit Delay4/1 -> AND1/2
  PHASE  4/5(docked) -> NOT6/1, Scope3/1, docked/1
  simulator of pings from sensor/1(# OF PINGS) -> "logic to recognize where i am, based on the # of pings"/1(num)
  NOT3/1 -> Subsystem3/Enable, end phase 3/Enable, AND1/1
  AND1/1 -> PHASE  3/Enable
  OR/1 -> Scope11/1, AND2/1
  PHASE  3/2(s_p_auv) -> Switch1/3, ship_p_auv1/1
  PHASE  4/3(s_psi_auv) -> ship_psi_auv2/1
  NOT5/1 -> Unit Delay4/1
  PHASE  3/3(s_psi_auv) -> Switch/3, ship_psi_auv1/1
  end phase 3/1(num_of_pings) -> Subsystem3/1(num)
  AND2/1 -> Scope10/1, PHASE  4/Enable
  stop_flag/1 -> simulator of pings from sensor/2(stop_flag)
  ds_p_auv/1 -> simulator of pings from sensor/1(ds_p_auv)
  ship_p_auv/1 -> Switch1/1, PHASE  3/2(ship_p_auv)
  PHASE  4/1(speed body) -> speed body1/1
  PHASE  3/1(speed body) -> speed body/1
  NOT6/1 -> Unit Delay5/1
  Switch/1 -> PHASE  4/1(ship_psi_auv)
  Switch1/1 -> PHASE  4/2(ship_p_auv)
  PHASE  3/5(state_est_plane) -> state_est_plane_3/1
  PHASE  3/6(state_est_z) -> state_est_z_3/1
  PHASE  4/6(state_est_plane) -> state_est_plane_4/1
  PHASE  4/7(state_est_z) -> state_est_z_4/1
  PHASE  4/8(state_est_yaw_rel_error) -> state_est_yaw_rel_4/1
  PHASE  4/9(ds_p_auv_phase4) -> ds_p_auv_phase4/1
  PHASE  3/7(error speed) -> error speed/1
  PHASE  3/9("z_err ") -> "z_err "/1
  PHASE  3/8(yaw_error) -> yaw_error/1
  PHASE  4/10(error speed) -> error speed 4/1
  PHASE  4/11(angle) -> angle 4/1
  PHASE  4/12("z_err ") -> z err 4/1

== system simulation_environment_thesis/toWorkspace blocks for plotting ==
  state : Inport
  psi1 : Inport  Port=2
  body_speed_1 : Inport  Port=3
  psi2 : Inport  Port=4
  p1 : Inport  Port=5
  p2 : Inport  Port=6
  speed body2 : Inport  Port=7
  speed body3 : Inport  Port=8
  psi3 : Inport  Port=9
  p3 : Inport  Port=10
  speed_body4 : Inport  Port=11
  psi4 : Inport  Port=12
  p4 : Inport  Port=13
  Demux{,1..2,6} (4 alike) : Demux
  Demux{3..5,7} (4 alike) : Demux  Outputs=3
  Multiport Switch{,1..6} (7 alike) : MultiPortSwitch  Inputs=4  SaturateOnIntegerOverflow=off  InputSameDT=off
  To Workspace : ToWorkspace  VariableName=z  SampleTime=-1
  To Workspace1 : ToWorkspace  VariableName=w  SampleTime=-1
  To Workspace15 : ToWorkspace  VariableName=x  SampleTime=-1
  To Workspace16 : ToWorkspace  VariableName=y  SampleTime=-1
  To Workspace17 : ToWorkspace  VariableName=u  SampleTime=-1
  To Workspace18 : ToWorkspace  VariableName=v  SampleTime=-1
  To Workspace19 : ToWorkspace  VariableName=yaw  SampleTime=-1
  wires:
  Multiport Switch/1 -> To Workspace19/1
  Multiport Switch5/1 -> To Workspace16/1
  Multiport Switch2/1 -> To Workspace18/1
  Multiport Switch1/1 -> To Workspace17/1
  Multiport Switch6/1 -> To Workspace/1
  Multiport Switch3/1 -> To Workspace1/1
  psi1/1 -> Multiport Switch/2
  psi2/1 -> Multiport Switch/3
  psi3/1 -> Multiport Switch/4
  body_speed_1/1 -> Demux/1
  speed body2/1 -> Demux1/1
  speed body3/1 -> Demux2/1
  Demux/1 -> Multiport Switch1/2
  Demux1/1 -> Multiport Switch1/3
  Demux2/1 -> Multiport Switch1/4
  Demux/2 -> Multiport Switch2/2
  Demux1/2 -> Multiport Switch2/3
  Demux2/2 -> Multiport Switch2/4
  Demux/3 -> Multiport Switch3/2
  Demux1/3 -> Multiport Switch3/3
  Demux2/3 -> Multiport Switch3/4
  Demux3/1 -> Multiport Switch4/2
  Demux3/2 -> Multiport Switch5/2
  Demux4/2 -> Multiport Switch5/3
  p3/1 -> Demux5/1
  Demux5/2 -> Multiport Switch5/4
  Demux3/3 -> Multiport Switch6/2
  Demux5/1 -> Multiport Switch4/4
  Multiport Switch4/1 -> To Workspace15/1
  Demux4/3 -> Multiport Switch6/3
  state/1 -> Multiport Switch3/1, Multiport Switch2/1, Multiport Switch1/1, Multiport Switch/1, Multiport Switch6/1, Multiport Switch5/1, Multiport Switch4/1
  p1/1 -> Demux3/1
  p2/1 -> Demux4/1
  Demux4/1 -> Multiport Switch4/3
  Demux5/3 -> Multiport Switch6/4
  speed_body4/1 -> Demux6/1
  psi4/1 -> Multiport Switch/5
  p4/1 -> Demux7/1
  Demux6/1 -> Multiport Switch1/5
  Demux6/2 -> Multiport Switch2/5
  Demux6/3 -> Multiport Switch3/5
  Demux7/1 -> Multiport Switch4/5
  Demux7/2 -> Multiport Switch5/5
  Demux7/3 -> Multiport Switch6/5

== system simulation_environment_thesis/toWorkspace blocks for statistical analysis on the error wrt the references ==
  state : Inport
  psi1 : Inport  Port=2
  body_speed_1 : Inport  Port=3
  psi2 : Inport  Port=4
  p1 : Inport  Port=5
  p2 : Inport  Port=6
  speed body2 : Inport  Port=7
  speed body3 : Inport  Port=8
  psi3 : Inport  Port=9
  p3 : Inport  Port=10
  speed_body4 : Inport  Port=11
  psi4 : Inport  Port=12
  p4 : Inport  Port=13
  Demux{,1..2,6} (4 alike) : Demux
  Multiport Switch{,1..4,6} (6 alike) : MultiPortSwitch  Inputs=4  SaturateOnIntegerOverflow=off  InputSameDT=off
  To Workspace : ToWorkspace  VariableName=z_error  SampleTime=-1
  To Workspace1 : ToWorkspace  VariableName=w_error  SampleTime=-1
  To Workspace17 : ToWorkspace  VariableName=u_error  SampleTime=-1
  To Workspace18 : ToWorkspace  VariableName=v_error  SampleTime=-1
  To Workspace19 : ToWorkspace  VariableName=yaw_error  SampleTime=-1
  To Workspace2 : ToWorkspace  VariableName=r_error  SampleTime=-1
  wires:
  Multiport Switch/1 -> To Workspace19/1
  Multiport Switch2/1 -> To Workspace18/1
  Multiport Switch1/1 -> To Workspace17/1
  Multiport Switch6/1 -> To Workspace/1
  Multiport Switch3/1 -> To Workspace1/1
  psi1/1 -> Multiport Switch/2
  psi2/1 -> Multiport Switch/3
  psi3/1 -> Multiport Switch/4
  body_speed_1/1 -> Demux/1
  speed body2/1 -> Demux1/1
  speed body3/1 -> Demux2/1
  Demux/1 -> Multiport Switch1/2
  Demux1/1 -> Multiport Switch1/3
  Demux2/1 -> Multiport Switch1/4
  Demux/2 -> Multiport Switch2/2
  Demux1/2 -> Multiport Switch2/3
  Demux2/2 -> Multiport Switch2/4
  Demux/3 -> Multiport Switch3/2
  Demux1/3 -> Multiport Switch3/3
  Demux2/3 -> Multiport Switch3/4
  p1/1 -> Multiport Switch6/2
  p2/1 -> Multiport Switch6/3
  state/1 -> Multiport Switch4/1, Multiport Switch3/1, Multiport Switch2/1, Multiport Switch1/1, Multiport Switch/1, Multiport Switch6/1
  p3/1 -> Multiport Switch6/4
  speed_body4/1 -> Demux6/1
  psi4/1 -> Multiport Switch/5
  Demux6/1 -> Multiport Switch1/5
  Demux6/2 -> Multiport Switch2/5
  Demux6/3 -> Multiport Switch3/5
  p4/1 -> Multiport Switch6/5
  Multiport Switch4/1 -> To Workspace2/1
  Demux/4 -> Multiport Switch4/2
  Demux1/4 -> Multiport Switch4/3
  Demux2/4 -> Multiport Switch4/4
  Demux6/4 -> Multiport Switch4/5

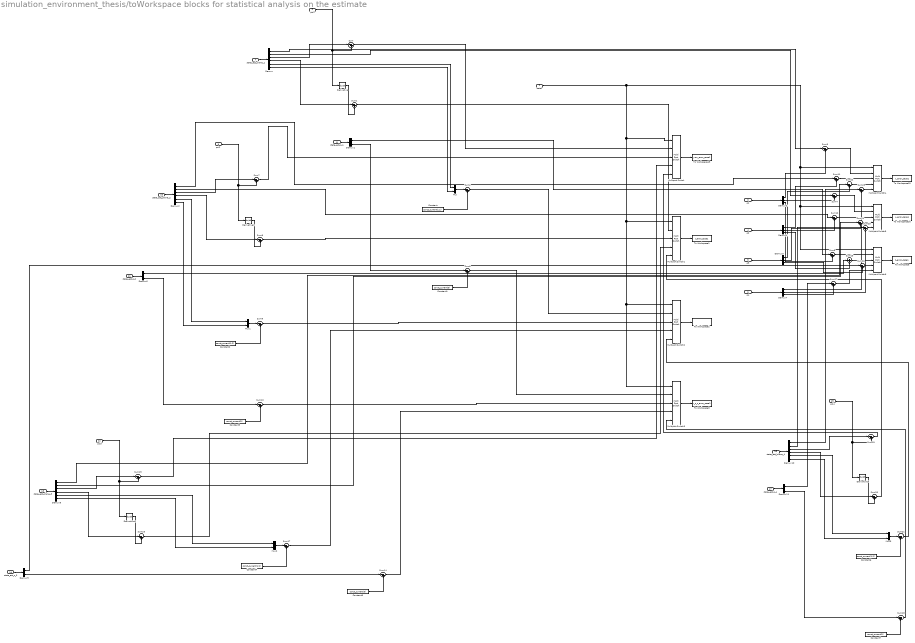
== system simulation_environment_thesis/toWorkspace blocks for statistical analysis on the estimate   ==
  state : Inport
  psi1 : Inport  Port=2
  p1 : Inport  Port=3
  p2 : Inport  Port=4
  p3 : Inport  Port=5
  p4 : Inport  Port=6
  state_est_plane_1 : Inport  Port=7
  state_est_z_1 : Inport  Port=8
  psi2 : Inport  Port=9
  state_est_plane_2 : Inport  Port=10
  state_est_z_2 : Inport  Port=11
  psi3 : Inport  Port=12
  state_est_plane_3 : Inport  Port=13
  state_est_z_3 : Inport  Port=14
  psi4 : Inport  Port=15
  state_est_plane_4 : Inport  Port=16
  state_est_z_4 : Inport  Port=17
  Constant{,2,4,6} (4 alike) : Constant  Value=const_current(1:2)
  Constant{1,3,5,7} (4 alike) : Constant  Value=const_current(3)
  Demux{,2,8,10} (4 alike) : Demux  Outputs=6
  Demux{1,6,9,11} (4 alike) : Demux  Outputs=2
  Demux{3..5,7} (4 alike) : Demux  Outputs=3
  Derivative{,1..3} (4 alike) : Derivative
  Multiport Switch{,1..6} (7 alike) : MultiPortSwitch  Inputs=4  SaturateOnIntegerOverflow=off  InputSameDT=off
  Mux{,1..3} (4 alike) : Mux  Inputs=2
  Sum{,1..27} (28 alike) : Sum  Inputs=|-+
  To Workspace : ToWorkspace  VariableName=z_error_case1  SampleTime=-1
  To Workspace1 : ToWorkspace  VariableName=r_error_case1  SampleTime=-1
  To Workspace15 : ToWorkspace  VariableName=x_error_case1  SampleTime=-1
  To Workspace16 : ToWorkspace  VariableName=y_error_case1  SampleTime=-1
  To Workspace19 : ToWorkspace  VariableName=yaw_error_case1  SampleTime=-1
  To Workspace2 : ToWorkspace  VariableName=v_c_x_y_error_case1  SampleTime=-1
  To Workspace3 : ToWorkspace  VariableName=v_c_z_error_case1  SampleTime=-1
  wires:
  Multiport Switch/1 -> To Workspace19/1
  Multiport Switch5/1 -> To Workspace16/1
  Multiport Switch6/1 -> To Workspace/1
  Demux3/1 -> Sum3/2
  p3/1 -> Demux5/1
  Multiport Switch4/1 -> To Workspace15/1
  p1/1 -> Demux3/1
  p2/1 -> Demux4/1
  p4/1 -> Demux7/1
  state_est_plane_1/1 -> Demux/1
  Demux/3 -> Sum/1
  psi1/1 -> Derivative/1, Sum/2
  Sum/1 -> Multiport Switch/2
  Multiport Switch1/1 -> To Workspace1/1
  Derivative/1 -> Sum1/2
  Demux/4 -> Sum1/1
  Sum1/1 -> Multiport Switch1/2
  Sum2/1 -> Multiport Switch2/2
  Multiport Switch2/1 -> To Workspace2/1
  Mux/1 -> Sum2/1
  Demux/5 -> Mux/1
  Demux/6 -> Mux/2
  Constant/1 -> Sum2/2
  Demux/1 -> Sum3/1
  Sum3/1 -> Multiport Switch4/2
  Demux3/2 -> Sum4/2
  Demux/2 -> Sum4/1
  Sum4/1 -> Multiport Switch5/2
  Demux3/3 -> Sum5/2
  state_est_z_1/1 -> Demux1/1
  Demux1/1 -> Sum5/1
  Sum5/1 -> Multiport Switch6/2
  Multiport Switch3/1 -> To Workspace3/1
  Sum6/1 -> Multiport Switch3/2
  state/1 -> Multiport Switch1/1, Multiport Switch3/1, Multiport Switch2/1, Multiport Switch/1, Multiport Switch4/1, Multiport Switch6/1, Multiport Switch5/1
  Constant1/1 -> Sum6/2
  Demux1/2 -> Sum6/1
  Derivative1/1 -> Sum8/2
  Demux6/2 -> Sum10/1
  Demux2/5 -> Mux1/1
  Demux2/4 -> Sum8/1
  Constant3/1 -> Sum10/2
  Demux2/1 -> Sum11/1
  state_est_z_2/1 -> Demux6/1
  Sum7/1 -> Multiport Switch/3
  Demux2/3 -> Sum7/1
  Demux6/1 -> Sum13/1
  Demux2/6 -> Mux1/2
  psi2/1 -> Derivative1/1, Sum7/2
  Constant2/1 -> Sum9/2
  Sum9/1 -> Multiport Switch2/3
  Sum10/1 -> Multiport Switch3/3
  Sum8/1 -> Multiport Switch1/3
  Mux1/1 -> Sum9/1
  state_est_plane_2/1 -> Demux2/1
  Demux2/2 -> Sum12/1
  Sum11/1 -> Multiport Switch4/3
  Demux4/1 -> Sum11/2
  Sum12/1 -> Multiport Switch5/3
  Demux4/2 -> Sum12/2
  Demux4/3 -> Sum13/2
  Sum13/1 -> Multiport Switch6/3
  Constant5/1 -> Sum14/2
  Demux8/4 -> Sum16/1
  Demux9/2 -> Sum14/1
  Demux8/5 -> Mux2/1
  Derivative2/1 -> Sum16/2
  state_est_plane_3/1 -> Demux8/1
  Mux2/1 -> Sum17/1
  Demux8/6 -> Mux2/2
  Constant4/1 -> Sum17/2
  psi3/1 -> Derivative2/1, Sum15/2
  state_est_z_3/1 -> Demux9/1
  Demux8/3 -> Sum15/1
  Demux10/3 -> Sum19/1
  state_est_z_4/1 -> Demux11/1
  psi4/1 -> Derivative3/1, Sum19/2
  Demux10/6 -> Mux3/2
  state_est_plane_4/1 -> Demux10/1
  Constant6/1 -> Sum21/2
  Mux3/1 -> Sum21/1
  Derivative3/1 -> Sum20/2
  Demux10/4 -> Sum20/1
  Demux11/2 -> Sum18/1
  Demux10/5 -> Mux3/1
  Constant7/1 -> Sum18/2
  Sum15/1 -> Multiport Switch/4
  Sum16/1 -> Multiport Switch1/4
  Sum17/1 -> Multiport Switch2/4
  Sum14/1 -> Multiport Switch3/4
  Sum19/1 -> Multiport Switch/5
  Sum20/1 -> Multiport Switch1/5
  Sum21/1 -> Multiport Switch2/5
  Sum18/1 -> Multiport Switch3/5
  Sum22/1 -> Multiport Switch4/4
  Demux5/1 -> Sum22/2
  Demux8/1 -> Sum22/1
  Sum23/1 -> Multiport Switch4/5
  Demux7/1 -> Sum23/2
  Demux10/1 -> Sum23/1
  Sum24/1 -> Multiport Switch5/4
  Demux5/2 -> Sum24/2
  Demux8/2 -> Sum24/1
  Sum25/1 -> Multiport Switch5/5
  Demux7/2 -> Sum25/2
  Demux10/2 -> Sum25/1
  Sum26/1 -> Multiport Switch6/4
  Demux5/3 -> Sum26/2
  Demux9/1 -> Sum26/1
  Sum27/1 -> Multiport Switch6/5
  Demux7/3 -> Sum27/2
  Demux11/1 -> Sum27/1

== system simulation_environment_thesis/toWorkspace blocks 21 ==
  state : Inport
  psi1 : Inport  Port=2
  body_speed_1 : Inport  Port=3
  psi2 : Inport  Port=4
  p1 : Inport  Port=5
  p2 : Inport  Port=6
  speed body2 : Inport  Port=7
  speed body3 : Inport  Port=8
  psi3 : Inport  Port=9
  p3 : Inport  Port=10
  speed_body4 : Inport  Port=11
  psi4 : Inport  Port=12
  p4 : Inport  Port=13
  Demux{,1..2,6} (4 alike) : Demux
  Demux{3..5,7} (4 alike) : Demux  Outputs=3
  Multiport Switch{,1..6} (7 alike) : MultiPortSwitch  Inputs=4  SaturateOnIntegerOverflow=off  InputSameDT=off
  To Workspace : ToWorkspace  VariableName=z  SampleTime=-1
  To Workspace1 : ToWorkspace  VariableName=w  SampleTime=-1
  To Workspace15 : ToWorkspace  VariableName=x  SampleTime=-1
  To Workspace16 : ToWorkspace  VariableName=y  SampleTime=-1
  To Workspace17 : ToWorkspace  VariableName=u  SampleTime=-1
  To Workspace18 : ToWorkspace  VariableName=v  SampleTime=-1
  To Workspace19 : ToWorkspace  VariableName=yaw  SampleTime=-1
  wires:
  Multiport Switch/1 -> To Workspace19/1
  Multiport Switch5/1 -> To Workspace16/1
  Multiport Switch2/1 -> To Workspace18/1
  Multiport Switch1/1 -> To Workspace17/1
  Multiport Switch6/1 -> To Workspace/1
  Multiport Switch3/1 -> To Workspace1/1
  psi1/1 -> Multiport Switch/2
  psi2/1 -> Multiport Switch/3
  psi3/1 -> Multiport Switch/4
  body_speed_1/1 -> Demux/1
  speed body2/1 -> Demux1/1
  speed body3/1 -> Demux2/1
  Demux/1 -> Multiport Switch1/2
  Demux1/1 -> Multiport Switch1/3
  Demux2/1 -> Multiport Switch1/4
  Demux/2 -> Multiport Switch2/2
  Demux1/2 -> Multiport Switch2/3
  Demux2/2 -> Multiport Switch2/4
  Demux/3 -> Multiport Switch3/2
  Demux1/3 -> Multiport Switch3/3
  Demux2/3 -> Multiport Switch3/4
  Demux3/1 -> Multiport Switch4/2
  Demux3/2 -> Multiport Switch5/2
  Demux4/2 -> Multiport Switch5/3
  p3/1 -> Demux5/1
  Demux5/2 -> Multiport Switch5/4
  Demux3/3 -> Multiport Switch6/2
  Demux5/1 -> Multiport Switch4/4
  Multiport Switch4/1 -> To Workspace15/1
  Demux4/3 -> Multiport Switch6/3
  state/1 -> Multiport Switch3/1, Multiport Switch2/1, Multiport Switch1/1, Multiport Switch/1, Multiport Switch6/1, Multiport Switch5/1, Multiport Switch4/1
  p1/1 -> Demux3/1
  p2/1 -> Demux4/1
  Demux4/1 -> Multiport Switch4/3
  Demux5/3 -> Multiport Switch6/4
  speed_body4/1 -> Demux6/1
  psi4/1 -> Multiport Switch/5
  p4/1 -> Demux7/1
  Demux6/1 -> Multiport Switch1/5
  Demux6/2 -> Multiport Switch2/5
  Demux6/3 -> Multiport Switch3/5
  Demux7/1 -> Multiport Switch4/5
  Demux7/2 -> Multiport Switch5/5
  Demux7/3 -> Multiport Switch6/5

== system simulation_environment_thesis/toWorkspace blocks 22 ==
  state : Inport
  psi1 : Inport  Port=2
  body_speed_1 : Inport  Port=3
  psi2 : Inport  Port=4
  p1 : Inport  Port=5
  p2 : Inport  Port=6
  speed body2 : Inport  Port=7
  speed body3 : Inport  Port=8
  psi3 : Inport  Port=9
  p3 : Inport  Port=10
  speed_body4 : Inport  Port=11
  psi4 : Inport  Port=12
  p4 : Inport  Port=13
  Demux{,1..2,6} (4 alike) : Demux
  Demux{3..5,7} (4 alike) : Demux  Outputs=3
  Multiport Switch{,1..6} (7 alike) : MultiPortSwitch  Inputs=4  SaturateOnIntegerOverflow=off  InputSameDT=off
  To Workspace : ToWorkspace  VariableName=z  SampleTime=-1
  To Workspace1 : ToWorkspace  VariableName=w  SampleTime=-1
  To Workspace15 : ToWorkspace  VariableName=x  SampleTime=-1
  To Workspace16 : ToWorkspace  VariableName=y  SampleTime=-1
  To Workspace17 : ToWorkspace  VariableName=u  SampleTime=-1
  To Workspace18 : ToWorkspace  VariableName=v  SampleTime=-1
  To Workspace19 : ToWorkspace  VariableName=yaw  SampleTime=-1
  wires:
  Multiport Switch/1 -> To Workspace19/1
  Multiport Switch5/1 -> To Workspace16/1
  Multiport Switch2/1 -> To Workspace18/1
  Multiport Switch1/1 -> To Workspace17/1
  Multiport Switch6/1 -> To Workspace/1
  Multiport Switch3/1 -> To Workspace1/1
  psi1/1 -> Multiport Switch/2
  psi2/1 -> Multiport Switch/3
  psi3/1 -> Multiport Switch/4
  body_speed_1/1 -> Demux/1
  speed body2/1 -> Demux1/1
  speed body3/1 -> Demux2/1
  Demux/1 -> Multiport Switch1/2
  Demux1/1 -> Multiport Switch1/3
  Demux2/1 -> Multiport Switch1/4
  Demux/2 -> Multiport Switch2/2
  Demux1/2 -> Multiport Switch2/3
  Demux2/2 -> Multiport Switch2/4
  Demux/3 -> Multiport Switch3/2
  Demux1/3 -> Multiport Switch3/3
  Demux2/3 -> Multiport Switch3/4
  Demux3/1 -> Multiport Switch4/2
  Demux3/2 -> Multiport Switch5/2
  Demux4/2 -> Multiport Switch5/3
  p3/1 -> Demux5/1
  Demux5/2 -> Multiport Switch5/4
  Demux3/3 -> Multiport Switch6/2
  Demux5/1 -> Multiport Switch4/4
  Multiport Switch4/1 -> To Workspace15/1
  Demux4/3 -> Multiport Switch6/3
  state/1 -> Multiport Switch3/1, Multiport Switch2/1, Multiport Switch1/1, Multiport Switch/1, Multiport Switch6/1, Multiport Switch5/1, Multiport Switch4/1
  p1/1 -> Demux3/1
  p2/1 -> Demux4/1
  Demux4/1 -> Multiport Switch4/3
  Demux5/3 -> Multiport Switch6/4
  speed_body4/1 -> Demux6/1
  psi4/1 -> Multiport Switch/5
  p4/1 -> Demux7/1
  Demux6/1 -> Multiport Switch1/5
  Demux6/2 -> Multiport Switch2/5
  Demux6/3 -> Multiport Switch3/5
  Demux7/1 -> Multiport Switch4/5
  Demux7/2 -> Multiport Switch5/5
  Demux7/3 -> Multiport Switch6/5
